simulink model "ofdm_txrx_supermimo_coded"  (Simulink 7.1) (continued)
== system ofdm_txrx_supermimo_coded/EDK Processor == (continued)
      |                                      RNWReg, ...
      |                                      bankAddr, ...
      |                                      xl_slice(linearAddr, ...
      |                                               xl_nbits(linearAddr) - 1, ...
      |                                               5) ...
      |                                     );
      | if opCode_sm_PktBufFreqOffsets == xl_concat(xfix({xlUnsigned, 4, 0}, 8), ...
      |                                          xfix({xlUnsigned, ...
      |                                                xl_nbits(linearAddr) - 5, ...
      |                                                0}, ...
      |                                                66) ...
      |                                         );
      |     sm_PktBufFreqOffsets_we_reg = true;
      | else
      |     sm_PktBufFreqOffsets_we_reg = false;
      | end
      | persistent sm_RxModulation_we_reg; sm_RxModulation_we_reg = xl_state(false, {xlBoolean});
      | sm_RxModulation_we = sm_RxModulation_we_reg;
      | opCode_sm_RxModulation = xl_concat(addrAck, ...
      |                                      RNWReg, ...
      |                                      bankAddr, ...
      |                                      xl_slice(linearAddr, ...
      |                                               xl_nbits(linearAddr) - 1, ...
      |                                               8) ...
      |                                     );
      | if opCode_sm_RxModulation == xl_concat(xfix({xlUnsigned, 4, 0}, 8), ...
      |                                          xfix({xlUnsigned, ...
      |                                                xl_nbits(linearAddr) - 8, ...
      |                                                0}, ...
      |                                                6) ...
      |                                         );
      |     sm_RxModulation_we_reg = true;
      | else
      |     sm_RxModulation_we_reg = false;
      | end
      | persistent sm_TxHeaderTranslate_we_reg; sm_TxHeaderTranslate_we_reg = xl_state(false, {xlBoolean});
      | sm_TxHeaderTranslate_we = sm_TxHeaderTranslate_we_reg;
      | opCode_sm_TxHeaderTranslate = xl_concat(addrAck, ...
      |                                      RNWReg, ...
      |                                      bankAddr, ...
      |                                      xl_slice(linearAddr, ...
      |                                               xl_nbits(linearAddr) - 1, ...
      |                                               10) ...
      |                                     );
      | if opCode_sm_TxHeaderTranslate == xl_concat(xfix({xlUnsigned, 4, 0}, 8), ...
      |                                          xfix({xlUnsigned, ...
      |                                                xl_nbits(linearAddr) - 10, ...
      |                                                0}, ...
      |                                                0) ...
      |                                         );
      |     sm_TxHeaderTranslate_we_reg = true;
      | else
      |     sm_TxHeaderTranslate_we_reg = false;
      | end
      | persistent sm_TxModulation_we_reg; sm_TxModulation_we_reg = xl_state(false, {xlBoolean});
      | sm_TxModulation_we = sm_TxModulation_we_reg;
      | opCode_sm_TxModulation = xl_concat(addrAck, ...
      |                                      RNWReg, ...
      |                                      bankAddr, ...
      |                                      xl_slice(linearAddr, ...
      |                                               xl_nbits(linearAddr) - 1, ...
      |                                               8) ...
      |                                     );
      | if opCode_sm_TxModulation == xl_concat(xfix({xlUnsigned, 4, 0}, 8), ...
      |                                          xfix({xlUnsigned, ...
      |                                                xl_nbits(linearAddr) - 8, ...
      |                                                0}, ...
      |                                                7) ...
      |                                         );
      |     sm_TxModulation_we_reg = true;
      | else
      |     sm_TxModulation_we_reg = false;
      | end
      | 
      | 
      | % 'addr' ports of 'Shared Memory' blocks
      | persistent sm_ChannelEstimates_addr_reg; 
      | sm_ChannelEstimates_addr_reg = xl_state(0, {xlUnsigned, 8, 0});
      | sm_ChannelEstimates_addr = sm_ChannelEstimates_addr_reg;
      | if addrAck == 1
      |     sm_ChannelEstimates_addr_reg = xl_slice(linearAddr, 8, 0);
      | else
      |     sm_ChannelEstimates_addr_reg = sm_ChannelEstimates_addr_reg;
      | end
      | persistent sm_EVM_perSC_addr_reg; 
      | sm_EVM_perSC_addr_reg = xl_state(0, {xlUnsigned, 6, 0});
      | sm_EVM_perSC_addr = sm_EVM_perSC_addr_reg;
      | if addrAck == 1
      |     sm_EVM_perSC_addr_reg = xl_slice(linearAddr, 6, 0);
      | else
      |     sm_EVM_perSC_addr_reg = sm_EVM_perSC_addr_reg;
      | end
      | persistent sm_EVM_perSym_addr_reg; 
      | sm_EVM_perSym_addr_reg = xl_state(0, {xlUnsigned, 8, 0});
      | sm_EVM_perSym_addr = sm_EVM_perSym_addr_reg;
      | if addrAck == 1
      |     sm_EVM_perSym_addr_reg = xl_slice(linearAddr, 8, 0);
      | else
      |     sm_EVM_perSym_addr_reg = sm_EVM_perSym_addr_reg;
      | end
      | persistent sm_PktBufFreqOffsets_addr_reg; 
      | sm_PktBufFreqOffsets_addr_reg = xl_state(0, {xlUnsigned, 5, 0});
      | sm_PktBufFreqOffsets_addr = sm_PktBufFreqOffsets_addr_reg;
      | if addrAck == 1
      |     sm_PktBufFreqOffsets_addr_reg = xl_slice(linearAddr, 5, 0);
      | else
      |     sm_PktBufFreqOffsets_addr_reg = sm_PktBufFreqOffsets_addr_reg;
      | end
      | persistent sm_RxModulation_addr_reg; 
      | sm_RxModulation_addr_reg = xl_state(0, {xlUnsigned, 8, 0});
      | sm_RxModulation_addr = sm_RxModulation_addr_reg;
      | if addrAck == 1
      |     sm_RxModulation_addr_reg = xl_slice(linearAddr, 8, 0);
      | else
      |     sm_RxModulation_addr_reg = sm_RxModulation_addr_reg;
      | end
      | persistent sm_TxHeaderTranslate_addr_reg; 
      | sm_TxHeaderTranslate_addr_reg = xl_state(0, {xlUnsigned, 10, 0});
      | sm_TxHeaderTranslate_addr = sm_TxHeaderTranslate_addr_reg;
      | if addrAck == 1
      |     sm_TxHeaderTranslate_addr_reg = xl_slice(linearAddr, 10, 0);
      | else
      |     sm_TxHeaderTranslate_addr_reg = sm_TxHeaderTranslate_addr_reg;
      | end
      | persistent sm_TxModulation_addr_reg; 
      | sm_TxModulation_addr_reg = xl_state(0, {xlUnsigned, 8, 0});
      | sm_TxModulation_addr = sm_TxModulation_addr_reg;
      | if addrAck == 1
      |     sm_TxModulation_addr_reg = xl_slice(linearAddr, 8, 0);
      | else
      |     sm_TxModulation_addr_reg = sm_TxModulation_addr_reg;
      | end
      | 
      | 
      | % 're' ports of 'From FIFO' blocks
      | 
      | 
      | % 'en' ports of 'To Register' blocks
      | if opCode == xl_concat(xfix({xlUnsigned, 4, 0}, 10), ...
      |                        xfix({xlUnsigned, xl_nbits(linearAddr), 0}, 0))
      |     sm_FEC_Config_w_en = true;
      | else
      |     sm_FEC_Config_w_en = false;
      | end
      | if opCode == xl_concat(xfix({xlUnsigned, 4, 0}, 10), ...
      |                        xfix({xlUnsigned, xl_nbits(linearAddr), 0}, 1))
      |     sm_Rx_AF_Blanking_w_en = true;
      | else
      |     sm_Rx_AF_Blanking_w_en = false;
      | end
      | if opCode == xl_concat(xfix({xlUnsigned, 4, 0}, 10), ...
      |                        xfix({xlUnsigned, xl_nbits(linearAddr), 0}, 2))
      |     sm_Rx_AF_TxScaling_w_en = true;
      | else
      |     sm_Rx_AF_TxScaling_w_en = false;
      | end
      | if opCode == xl_concat(xfix({xlUnsigned, 4, 0}, 10), ...
      |                        xfix({xlUnsigned, xl_nbits(linearAddr), 0}, 3))
      |     sm_Rx_ChanEst_MinMag_w_en = true;
      | else
      |     sm_Rx_ChanEst_MinMag_w_en = false;
      | end
      | if opCode == xl_concat(xfix({xlUnsigned, 4, 0}, 10), ...
      |                        xfix({xlUnsigned, xl_nbits(linearAddr), 0}, 4))
      |     sm_Rx_Constellation_Scaling_w_en = true;
      | else
      |     sm_Rx_Constellation_Scaling_w_en = false;
      | end
      | if opCode == xl_concat(xfix({xlUnsigned, 4, 0}, 10), ...
      |                        xfix({xlUnsigned, xl_nbits(linearAddr), 0}, 5))
      |     sm_Rx_ControlBits_w_en = true;
      | else
      |     sm_Rx_ControlBits_w_en = false;
      | end
      | if opCode == xl_concat(xfix({xlUnsigned, 4, 0}, 10), ...
      |                        xfix({xlUnsigned, xl_nbits(linearAddr), 0}, 6))
      |     sm_Rx_FixedPktLen_w_en = true;
      | else
      |     sm_Rx_FixedPktLen_w_en = false;
      | end
      | if opCode == xl_concat(xfix({xlUnsigned, 4, 0}, 10), ...
      |                        xfix({xlUnsigned, xl_nbits(linearAddr), 0}, 7))
      |     sm_Rx_OFDM_SymbolCounts_w_en = true;
      | else
      |     sm_Rx_OFDM_SymbolCounts_w_en = false;
      | end
      | if opCode == xl_concat(xfix({xlUnsigned, 4, 0}, 10), ...
      |                        xfix({xlUnsigned, xl_nbits(linearAddr), 0}, 8))
      |     sm_Rx_PilotCalcParams_w_en = true;
      | else
      |     sm_Rx_PilotCalcParams_w_en = false;
      | end
      | if opCode == xl_concat(xfix({xlUnsigned, 4, 0}, 10), ...
      |                        xfix({xlUnsigned, xl_nbits(linearAddr), 0}, 9))
      |     sm_Rx_PktDet_Delay_w_en = true;
      | else
      |     sm_Rx_PktDet_Delay_w_en = false;
      | end
      | if opCode == xl_concat(xfix({xlUnsigned, 4, 0}, 10), ...
      |                        xfix({xlUnsigned, xl_nbits(linearAddr), 0}, 10))
      |     sm_Rx_PktDet_LongCorr_Params_w_en = true;
      | else
      |     sm_Rx_PktDet_LongCorr_Params_w_en = false;
      | end
      | if opCode == xl_concat(xfix({xlUnsigned, 4, 0}, 10), ...
      |                        xfix({xlUnsigned, xl_nbits(linearAddr), 0}, 11))
      |     sm_Rx_PktDet_LongCorr_Thresholds_w_en = true;
      | else
      |     sm_Rx_PktDet_LongCorr_Thresholds_w_en = false;
      | end
      | if opCode == xl_concat(xfix({xlUnsigned, 4, 0}, 10), ...
      |                        xfix({xlUnsigned, xl_nbits(linearAddr), 0}, 12))
      |     sm_Rx_PreCFO_Options_w_en = true;
      | else
      |     sm_Rx_PreCFO_Options_w_en = false;
      | end
      | if opCode == xl_concat(xfix({xlUnsigned, 4, 0}, 10), ...
      |                        xfix({xlUnsigned, xl_nbits(linearAddr), 0}, 13))
      |     sm_Rx_PreCFO_PilotCalcCorrection_w_en = true;
      | else
      |     sm_Rx_PreCFO_PilotCalcCorrection_w_en = false;
      | end
      | if opCode == xl_concat(xfix({xlUnsigned, 4, 0}, 10), ...
      |                        xfix({xlUnsigned, xl_nbits(linearAddr), 0}, 14))
      |     sm_Rx_coarseCFO_correction_w_en = true;
      | else
      |     sm_Rx_coarseCFO_correction_w_en = false;
      | end
      | if opCode == xl_concat(xfix({xlUnsigned, 4, 0}, 10), ...
      |                        xfix({xlUnsigned, xl_nbits(linearAddr), 0}, 15))
      |     sm_Rx_pktByteNums_w_en = true;
      | else
      |     sm_Rx_pktByteNums_w_en = false;
      | end
      | if opCode == xl_concat(xfix({xlUnsigned, 4, 0}, 10), ...
      |                        xfix({xlUnsigned, xl_nbits(linearAddr), 0}, 16))
      |     sm_TxRx_AutoReply_Action0_w_en = true;
      | else
      |     sm_TxRx_AutoReply_Action0_w_en = false;
      | end
      | if opCode == xl_concat(xfix({xlUnsigned, 4, 0}, 10), ...
      |                        xfix({xlUnsigned, xl_nbits(linearAddr), 0}, 17))
      |     sm_TxRx_AutoReply_Action1_w_en = true;
      | else
      |     sm_TxRx_AutoReply_Action1_w_en = false;
      | end
      | if opCode == xl_concat(xfix({xlUnsigned, 4, 0}, 10), ...
      |                        xfix({xlUnsigned, xl_nbits(linearAddr), 0}, 18))
      |     sm_TxRx_AutoReply_Action2_w_en = true;
      | else
      |     sm_TxRx_AutoReply_Action2_w_en = false;
      | end
      | if opCode == xl_concat(xfix({xlUnsigned, 4, 0}, 10), ...
      |                        xfix({xlUnsigned, xl_nbits(linearAddr), 0}, 19))
      |     sm_TxRx_AutoReply_Action3_w_en = true;
      | else
      |     sm_TxRx_AutoReply_Action3_w_en = false;
      | end
      | if opCode == xl_concat(xfix({xlUnsigned, 4, 0}, 10), ...
      |                        xfix({xlUnsigned, xl_nbits(linearAddr), 0}, 20))
      |     sm_TxRx_AutoReply_Action4_w_en = true;
      | else
      |     sm_TxRx_AutoReply_Action4_w_en = false;
      | end
      | if opCode == xl_concat(xfix({xlUnsigned, 4, 0}, 10), ...
      |                        xfix({xlUnsigned, xl_nbits(linearAddr), 0}, 21))
      |     sm_TxRx_AutoReply_Action5_w_en = true;
      | else
      |     sm_TxRx_AutoReply_Action5_w_en = false;
      | end
      | if opCode == xl_concat(xfix({xlUnsigned, 4, 0}, 10), ...
      |                        xfix({xlUnsigned, xl_nbits(linearAddr), 0}, 22))
      |     sm_TxRx_AutoReply_Match0_w_en = true;
      | else
      |     sm_TxRx_AutoReply_Match0_w_en = false;
      | end
      | if opCode == xl_concat(xfix({xlUnsigned, 4, 0}, 10), ...
      |                        xfix({xlUnsigned, xl_nbits(linearAddr), 0}, 23))
      |     sm_TxRx_AutoReply_Match1_w_en = true;
      | else
      |     sm_TxRx_AutoReply_Match1_w_en = false;
      | end
      | if opCode == xl_concat(xfix({xlUnsigned, 4, 0}, 10), ...
      |                        xfix({xlUnsigned, xl_nbits(linearAddr), 0}, 24))
      |     sm_TxRx_AutoReply_Match2_w_en = true;
      | else
      |     sm_TxRx_AutoReply_Match2_w_en = false;
      | end
      | if opCode == xl_concat(xfix({xlUnsigned, 4, 0}, 10), ...
      |                        xfix({xlUnsigned, xl_nbits(linearAddr), 0}, 25))
      |     sm_TxRx_AutoReply_Match3_w_en = true;
      | else
      |     sm_TxRx_AutoReply_Match3_w_en = false;
      | end
      | if opCode == xl_concat(xfix({xlUnsigned, 4, 0}, 10), ...
      |                        xfix({xlUnsigned, xl_nbits(linearAddr), 0}, 26))
      |     sm_TxRx_AutoReply_Match4_w_en = true;
      | else
      |     sm_TxRx_AutoReply_Match4_w_en = false;
      | end
      | if opCode == xl_concat(xfix({xlUnsigned, 4, 0}, 10), ...
      |                        xfix({xlUnsigned, xl_nbits(linearAddr), 0}, 27))
      |     sm_TxRx_AutoReply_Match5_w_en = true;
      | else
      |     sm_TxRx_AutoReply_Match5_w_en = false;
      | end
      | if opCode == xl_concat(xfix({xlUnsigned, 4, 0}, 10), ...
      |                        xfix({xlUnsigned, xl_nbits(linearAddr), 0}, 28))
      |     sm_TxRx_AutoReply_Match6_w_en = true;
      | else
      |     sm_TxRx_AutoReply_Match6_w_en = false;
      | end
      | if opCode == xl_concat(xfix({xlUnsigned, 4, 0}, 10), ...
      |                        xfix({xlUnsigned, xl_nbits(linearAddr), 0}, 29))
      |     sm_TxRx_AutoReply_Match7_w_en = true;
      | else
      |     sm_TxRx_AutoReply_Match7_w_en = false;
      | end
      | if opCode == xl_concat(xfix({xlUnsigned, 4, 0}, 10), ...
      |                        xfix({xlUnsigned, xl_nbits(linearAddr), 0}, 30))
      |     sm_TxRx_FFT_Scaling_w_en = true;
      | else
      |     sm_TxRx_FFT_Scaling_w_en = false;
      | end
      | if opCode == xl_concat(xfix({xlUnsigned, 4, 0}, 10), ...
      |                        xfix({xlUnsigned, xl_nbits(linearAddr), 0}, 31))
      |     sm_TxRx_Interrupt_PktBuf_Ctrl_w_en = true;
      | else
      |     sm_TxRx_Interrupt_PktBuf_Ctrl_w_en = false;
      | end
      | if opCode == xl_concat(xfix({xlUnsigned, 4, 0}, 10), ...
      |                        xfix({xlUnsigned, xl_nbits(linearAddr), 0}, 32))
      |     sm_TxRx_Pilots_Index_w_en = true;
      | else
      |     sm_TxRx_Pilots_Index_w_en = false;
      | end
      | if opCode == xl_concat(xfix({xlUnsigned, 4, 0}, 10), ...
      |                        xfix({xlUnsigned, xl_nbits(linearAddr), 0}, 33))
      |     sm_TxRx_Pilots_Values_w_en = true;
      | else
      |     sm_TxRx_Pilots_Values_w_en = false;
      | end
      | if opCode == xl_concat(xfix({xlUnsigned, 4, 0}, 10), ...
      |                        xfix({xlUnsigned, xl_nbits(linearAddr), 0}, 34))
      |     sm_Tx_ControlBits_w_en = true;
      | else
      |     sm_Tx_ControlBits_w_en = false;
      | end
      | if opCode == xl_concat(xfix({xlUnsigned, 4, 0}, 10), ...
      |                        xfix({xlUnsigned, xl_nbits(linearAddr), 0}, 35))
      |     sm_Tx_Delays_w_en = true;
      | else
      |     sm_Tx_Delays_w_en = false;
      | end
      | if opCode == xl_concat(xfix({xlUnsigned, 4, 0}, 10), ...
      |                        xfix({xlUnsigned, xl_nbits(linearAddr), 0}, 36))
      |     sm_Tx_OFDM_SymCounts_w_en = true;
      | else
      |     sm_Tx_OFDM_SymCounts_w_en = false;
      | end
      | if opCode == xl_concat(xfix({xlUnsigned, 4, 0}, 10), ...
      |                        xfix({xlUnsigned, xl_nbits(linearAddr), 0}, 37))
      |     sm_Tx_Scaling_w_en = true;
      | else
      |     sm_Tx_Scaling_w_en = false;
      | end
      | if opCode == xl_concat(xfix({xlUnsigned, 4, 0}, 10), ...
      |                        xfix({xlUnsigned, xl_nbits(linearAddr), 0}, 38))
      |     sm_Tx_Start_Reset_Control_w_en = true;
      | else
      |     sm_Tx_Start_Reset_Control_w_en = false;
      | end
      | if opCode == xl_concat(xfix({xlUnsigned, 4, 0}, 10), ...
      |                        xfix({xlUnsigned, xl_nbits(linearAddr), 0}, 39))
      |     sm_pktDet_autoCorrParams_w_en = true;
      | else
      |     sm_pktDet_autoCorrParams_w_en = false;
      | end
      | if opCode == xl_concat(xfix({xlUnsigned, 4, 0}, 10), ...
      |                        xfix({xlUnsigned, xl_nbits(linearAddr), 0}, 40))
      |     sm_pktDet_controlBits_w_en = true;
      | else
      |     sm_pktDet_controlBits_w_en = false;
      | end
      | if opCode == xl_concat(xfix({xlUnsigned, 4, 0}, 10), ...
      |                        xfix({xlUnsigned, xl_nbits(linearAddr), 0}, 41))
      |     sm_pktDet_durations_w_en = true;
      | else
      |     sm_pktDet_durations_w_en = false;
      | end
      | if opCode == xl_concat(xfix({xlUnsigned, 4, 0}, 10), ...
      |                        xfix({xlUnsigned, xl_nbits(linearAddr), 0}, 42))
      |     sm_pktDet_thresholds_w_en = true;
      | else
      |     sm_pktDet_thresholds_w_en = false;
      | end
      | 
      | 
      | % 'din' ports of 'To FIFO' blocks
      | 
      | 
      | % 'we' ports of 'To FIFO' blocks
      | 
      | 
      | % 'din' ports of 'To Register' blocks
      | sm_FEC_Config_w_din = xl_force(xl_slice(wrDBus, 32 - 1, 0), ...
      |                                  xlUnsigned, ...
      |                                  0);
      | sm_Rx_AF_Blanking_w_din = xl_force(xl_slice(wrDBus, 32 - 1, 0), ...
      |                                  xlUnsigned, ...
      |                                  0);
      | sm_Rx_AF_TxScaling_w_din = xl_force(xl_slice(wrDBus, 32 - 1, 0), ...
      |                                  xlUnsigned, ...
      |                                  0);
      | sm_Rx_ChanEst_MinMag_w_din = xl_force(xl_slice(wrDBus, 32 - 1, 0), ...
      |                                  xlUnsigned, ...
      |                                  0);
      | sm_Rx_Constellation_Scaling_w_din = xl_force(xl_slice(wrDBus, 32 - 1, 0), ...
      |                                  xlUnsigned, ...
      |                                  0);
      | sm_Rx_ControlBits_w_din = xl_force(xl_slice(wrDBus, 32 - 1, 0), ...
      |                                  xlUnsigned, ...
      |                                  0);
      | sm_Rx_FixedPktLen_w_din = xl_force(xl_slice(wrDBus, 32 - 1, 0), ...
      |                                  xlUnsigned, ...
      |                                  0);
      | sm_Rx_OFDM_SymbolCounts_w_din = xl_force(xl_slice(wrDBus, 32 - 1, 0), ...
      |                                  xlUnsigned, ...
      |                                  0);
      | sm_Rx_PilotCalcParams_w_din = xl_force(xl_slice(wrDBus, 32 - 1, 0), ...
      |                                  xlUnsigned, ...
      |                                  0);
      | sm_Rx_PktDet_Delay_w_din = xl_force(xl_slice(wrDBus, 32 - 1, 0), ...
      |                                  xlUnsigned, ...
      |                                  0);
      | sm_Rx_PktDet_LongCorr_Params_w_din = xl_force(xl_slice(wrDBus, 32 - 1, 0), ...
      |                                  xlUnsigned, ...
      |                                  0);
      | sm_Rx_PktDet_LongCorr_Thresholds_w_din = xl_force(xl_slice(wrDBus, 32 - 1, 0), ...
      |                                  xlUnsigned, ...
      |                                  0);
      | sm_Rx_PreCFO_Options_w_din = xl_force(xl_slice(wrDBus, 32 - 1, 0), ...
      |                                  xlUnsigned, ...
      |                                  0);
      | sm_Rx_PreCFO_PilotCalcCorrection_w_din = xl_force(xl_slice(wrDBus, 32 - 1, 0), ...
      |                                  xlUnsigned, ...
      |                                  0);
      | sm_Rx_coarseCFO_correction_w_din = xl_force(xl_slice(wrDBus, 32 - 1, 0), ...
      |                                  xlUnsigned, ...
      |                                  0);
      | sm_Rx_pktByteNums_w_din = xl_force(xl_slice(wrDBus, 32 - 1, 0), ...
      |                                  xlUnsigned, ...
      |                                  0);
      | sm_TxRx_AutoReply_Action0_w_din = xl_force(xl_slice(wrDBus, 32 - 1, 0), ...
      |                                  xlUnsigned, ...
      |                                  0);
      | sm_TxRx_AutoReply_Action1_w_din = xl_force(xl_slice(wrDBus, 32 - 1, 0), ...
      |                                  xlUnsigned, ...
      |                                  0);
      | sm_TxRx_AutoReply_Action2_w_din = xl_force(xl_slice(wrDBus, 32 - 1, 0), ...
      |                                  xlUnsigned, ...
      |                                  0);
      | sm_TxRx_AutoReply_Action3_w_din = xl_force(xl_slice(wrDBus, 32 - 1, 0), ...
      |                                  xlUnsigned, ...
      |                                  0);
      | sm_TxRx_AutoReply_Action4_w_din = xl_force(xl_slice(wrDBus, 32 - 1, 0), ...
      |                                  xlUnsigned, ...
      |                                  0);
      | sm_TxRx_AutoReply_Action5_w_din = xl_force(xl_slice(wrDBus, 32 - 1, 0), ...
      |                                  xlUnsigned, ...
      |                                  0);
      | sm_TxRx_AutoReply_Match0_w_din = xl_force(xl_slice(wrDBus, 32 - 1, 0), ...
      |                                  xlUnsigned, ...
      |                                  0);
      | sm_TxRx_AutoReply_Match1_w_din = xl_force(xl_slice(wrDBus, 32 - 1, 0), ...
      |                                  xlUnsigned, ...
      |                                  0);
      | sm_TxRx_AutoReply_Match2_w_din = xl_force(xl_slice(wrDBus, 32 - 1, 0), ...
      |                                  xlUnsigned, ...
      |                                  0);
      | sm_TxRx_AutoReply_Match3_w_din = xl_force(xl_slice(wrDBus, 32 - 1, 0), ...
      |                                  xlUnsigned, ...
      |                                  0);
      | sm_TxRx_AutoReply_Match4_w_din = xl_force(xl_slice(wrDBus, 32 - 1, 0), ...
      |                                  xlUnsigned, ...
      |                                  0);
      | sm_TxRx_AutoReply_Match5_w_din = xl_force(xl_slice(wrDBus, 32 - 1, 0), ...
      |                                  xlUnsigned, ...
      |                                  0);
      | sm_TxRx_AutoReply_Match6_w_din = xl_force(xl_slice(wrDBus, 32 - 1, 0), ...
      |                                  xlUnsigned, ...
      |                                  0);
      | sm_TxRx_AutoReply_Match7_w_din = xl_force(xl_slice(wrDBus, 32 - 1, 0), ...
      |                                  xlUnsigned, ...
      |                                  0);
      | sm_TxRx_FFT_Scaling_w_din = xl_force(xl_slice(wrDBus, 12 - 1, 0), ...
      |                                  xlUnsigned, ...
      |                                  0);
      | sm_TxRx_Interrupt_PktBuf_Ctrl_w_din = xl_force(xl_slice(wrDBus, 32 - 1, 0), ...
      |                                  xlUnsigned, ...
      |                                  0);
      | sm_TxRx_Pilots_Index_w_din = xl_force(xl_slice(wrDBus, 32 - 1, 0), ...
      |                                  xlUnsigned, ...
      |                                  0);
      | sm_TxRx_Pilots_Values_w_din = xl_force(xl_slice(wrDBus, 32 - 1, 0), ...
      |                                  xlUnsigned, ...
      |                                  0);
      | sm_Tx_ControlBits_w_din = xl_force(xl_slice(wrDBus, 32 - 1, 0), ...
      |                                  xlUnsigned, ...
      |                                  0);
      | sm_Tx_Delays_w_din = xl_force(xl_slice(wrDBus, 32 - 1, 0), ...
      |                                  xlUnsigned, ...
      |                                  0);
      | sm_Tx_OFDM_SymCounts_w_din = xl_force(xl_slice(wrDBus, 32 - 1, 0), ...
      |                                  xlUnsigned, ...
      |                                  0);
      | sm_Tx_Scaling_w_din = xl_force(xl_slice(wrDBus, 32 - 1, 0), ...
      |                                  xlUnsigned, ...
      |                                  0);
      | sm_Tx_Start_Reset_Control_w_din = xl_force(xl_slice(wrDBus, 32 - 1, 0), ...
      |                                  xlUnsigned, ...
      |                                  0);
      | sm_pktDet_autoCorrParams_w_din = xl_force(xl_slice(wrDBus, 32 - 1, 0), ...
      |                                  xlUnsigned, ...
      |                                  0);
      | sm_pktDet_controlBits_w_din = xl_force(xl_slice(wrDBus, 32 - 1, 0), ...
      |                                  xlUnsigned, ...
      |                                  0);
      | sm_pktDet_durations_w_din = xl_force(xl_slice(wrDBus, 32 - 1, 0), ...
      |                                  xlUnsigned, ...
      |                                  0);
      | sm_pktDet_thresholds_w_din = xl_force(xl_slice(wrDBus, 32 - 1, 0), ...
      |                                  xlUnsigned, ...
      |                                  0);
      | 
      | 
      | persistent read_bank_out_reg; read_bank_out_reg = xl_state(0, {xlUnsigned, 32, 0});
      | read_bank_out = read_bank_out_reg;
      | 
      | persistent bankAddr_reg; bankAddr_reg = xl_state(0, bankAddr);
      | 
      | if bankAddr_reg == 0
      |     % Bank 0: Shared Memories
      |     read_bank_out_reg = ram_bank_out;
      | elseif bankAddr_reg == 1
      |     % Bank 1: From/To FIFOs
      |     read_bank_out_reg =  0;
      | elseif bankAddr_reg == 2
      |     % Bank 2: From/To Registers
      |     read_bank_out_reg = reg_bank_out;
      | elseif bankAddr_reg == 3
      |     % Bank 3: Configuration Registers
      |     read_bank_out_reg = 0;
      | end
      | 
      | bankAddr_reg = bankAddr;
      settings: block_version=10.1.3
  sg_plb_addrpref : xbsIndex_r4/Gateway In  arith_type=Unsigned  n_bits=16  bin_pt=0  quantization="Round  (unbiased: +/- Inf)"  overflow=Saturate  period=xlGetSimulinkPeriod(gcb)  dbl_ovrd=off  timing_constraint=None  locs_specified=off  LOCs={}  block_type=gatewayin
      settings: infoedit="Gateway in block.  Converts inputs of type Simulink integer, double and fixed point to  Xilinx fixed point type.<P><P>Hardware notes:  In hardware these blocks become top level input ports."  sginterface={'Xilinx'=>{'jtaghwcosim'=>{'non_memory_mapped_port'=>'sg_plb_addrpref'}},'iopad'=>{'constraint'=>'#'}}  block_version=10.1.3
  wires:
  plb_decode/2 -> plb_memmap/5, Sl_addrAck/1  "Sl_addrAck"
  plb_decode/4 -> Sl_wrDAck/1, Sl_wrComp/1  "Sl_wrDAck"
  plb_memmap/108 -> Shared Memory6/3  "TxModulation_we"
  plb_memmap/107 -> Shared Memory6/2  "TxModulation_din"
  plb_memmap/106 -> Shared Memory6/1  "TxModulation_addr"
  plb_memmap/105 -> Shared Memory5/3  "TxHeaderTranslate_we"
  plb_memmap/104 -> Shared Memory5/2  "TxHeaderTranslate_din"
  plb_memmap/103 -> Shared Memory5/1  "TxHeaderTranslate_addr"
  plb_memmap/102 -> Shared Memory4/3  "RxModulation_we"
  plb_memmap/101 -> Shared Memory4/2  "RxModulation_din"
  plb_memmap/100 -> Shared Memory4/1  "RxModulation_addr"
  plb_memmap/99 -> Shared Memory3/3  "PktBufFreqOffsets_we"
  plb_memmap/98 -> Shared Memory3/2  "PktBufFreqOffsets_din"
  plb_memmap/97 -> Shared Memory3/1  "PktBufFreqOffsets_addr"
  plb_memmap/96 -> Shared Memory2/3  "EVM_perSym_we"
  plb_memmap/95 -> Shared Memory2/2  "EVM_perSym_din"
  plb_memmap/94 -> Shared Memory2/1  "EVM_perSym_addr"
  plb_memmap/93 -> Shared Memory1/3  "EVM_perSC_we"
  plb_memmap/92 -> Shared Memory1/2  "EVM_perSC_din"
  plb_memmap/91 -> Shared Memory1/1  "EVM_perSC_addr"
  plb_memmap/90 -> Shared Memory/3  "ChannelEstimates_we"
  plb_memmap/89 -> Shared Memory/2  "ChannelEstimates_din"
  plb_memmap/88 -> Shared Memory/1  "ChannelEstimates_addr"
  plb_memmap/87 -> To Register42/2  "pktDet_thresholds_w_en"
  plb_memmap/86 -> To Register42/1  "pktDet_thresholds_w_din"
  plb_memmap/85 -> To Register41/2  "pktDet_durations_w_en"
  plb_memmap/84 -> To Register41/1  "pktDet_durations_w_din"
  plb_memmap/83 -> To Register40/2  "pktDet_controlBits_w_en"
  plb_memmap/82 -> To Register40/1  "pktDet_controlBits_w_din"
  plb_memmap/81 -> To Register39/2  "pktDet_autoCorrParams_w_en"
  plb_memmap/80 -> To Register39/1  "pktDet_autoCorrParams_w_din"
  plb_memmap/79 -> To Register38/2  "Tx_Start_Reset_Control_w_en"
  plb_memmap/78 -> To Register38/1  "Tx_Start_Reset_Control_w_din"
  plb_memmap/77 -> To Register37/2  "Tx_Scaling_w_en"
  plb_memmap/76 -> To Register37/1  "Tx_Scaling_w_din"
  plb_memmap/75 -> To Register36/2  "Tx_OFDM_SymCounts_w_en"
  plb_memmap/74 -> To Register36/1  "Tx_OFDM_SymCounts_w_din"
  plb_memmap/73 -> To Register35/2  "Tx_Delays_w_en"
  plb_memmap/72 -> To Register35/1  "Tx_Delays_w_din"
  plb_memmap/71 -> To Register34/2  "Tx_ControlBits_w_en"
  plb_memmap/70 -> To Register34/1  "Tx_ControlBits_w_din"
  plb_memmap/69 -> To Register33/2  "TxRx_Pilots_Values_w_en"
  plb_memmap/68 -> To Register33/1  "TxRx_Pilots_Values_w_din"
  plb_memmap/67 -> To Register32/2  "TxRx_Pilots_Index_w_en"
  plb_memmap/66 -> To Register32/1  "TxRx_Pilots_Index_w_din"
  plb_memmap/65 -> To Register31/2  "TxRx_Interrupt_PktBuf_Ctrl_w_en"
  plb_memmap/64 -> To Register31/1  "TxRx_Interrupt_PktBuf_Ctrl_w_din"
  plb_memmap/63 -> To Register30/2  "TxRx_FFT_Scaling_w_en"
  plb_memmap/62 -> To Register30/1  "TxRx_FFT_Scaling_w_din"
  plb_memmap/61 -> To Register29/2  "TxRx_AutoReply_Match7_w_en"
  plb_memmap/60 -> To Register29/1  "TxRx_AutoReply_Match7_w_din"
  plb_memmap/59 -> To Register28/2  "TxRx_AutoReply_Match6_w_en"
  plb_memmap/58 -> To Register28/1  "TxRx_AutoReply_Match6_w_din"
  plb_memmap/57 -> To Register27/2  "TxRx_AutoReply_Match5_w_en"
  plb_memmap/56 -> To Register27/1  "TxRx_AutoReply_Match5_w_din"
  plb_memmap/55 -> To Register26/2  "TxRx_AutoReply_Match4_w_en"
  plb_memmap/54 -> To Register26/1  "TxRx_AutoReply_Match4_w_din"
  plb_memmap/53 -> To Register25/2  "TxRx_AutoReply_Match3_w_en"
  plb_memmap/52 -> To Register25/1  "TxRx_AutoReply_Match3_w_din"
  plb_memmap/51 -> To Register24/2  "TxRx_AutoReply_Match2_w_en"
  plb_memmap/50 -> To Register24/1  "TxRx_AutoReply_Match2_w_din"
  plb_memmap/49 -> To Register23/2  "TxRx_AutoReply_Match1_w_en"
  plb_memmap/48 -> To Register23/1  "TxRx_AutoReply_Match1_w_din"
  plb_memmap/47 -> To Register22/2  "TxRx_AutoReply_Match0_w_en"
  plb_memmap/46 -> To Register22/1  "TxRx_AutoReply_Match0_w_din"
  plb_memmap/45 -> To Register21/2  "TxRx_AutoReply_Action5_w_en"
  plb_memmap/44 -> To Register21/1  "TxRx_AutoReply_Action5_w_din"
  plb_memmap/43 -> To Register20/2  "TxRx_AutoReply_Action4_w_en"
  plb_memmap/42 -> To Register20/1  "TxRx_AutoReply_Action4_w_din"
  plb_memmap/41 -> To Register19/2  "TxRx_AutoReply_Action3_w_en"
  plb_memmap/40 -> To Register19/1  "TxRx_AutoReply_Action3_w_din"
  plb_memmap/39 -> To Register18/2  "TxRx_AutoReply_Action2_w_en"
  plb_memmap/38 -> To Register18/1  "TxRx_AutoReply_Action2_w_din"
  plb_memmap/37 -> To Register17/2  "TxRx_AutoReply_Action1_w_en"
  plb_memmap/36 -> To Register17/1  "TxRx_AutoReply_Action1_w_din"
  plb_memmap/35 -> To Register16/2  "TxRx_AutoReply_Action0_w_en"
  plb_memmap/34 -> To Register16/1  "TxRx_AutoReply_Action0_w_din"
  plb_memmap/33 -> To Register15/2  "Rx_pktByteNums_w_en"
  plb_memmap/32 -> To Register15/1  "Rx_pktByteNums_w_din"
  plb_memmap/31 -> To Register14/2  "Rx_coarseCFO_correction_w_en"
  plb_memmap/30 -> To Register14/1  "Rx_coarseCFO_correction_w_din"
  plb_memmap/29 -> To Register13/2  "Rx_PreCFO_PilotCalcCorrection_w_en"
  plb_memmap/28 -> To Register13/1  "Rx_PreCFO_PilotCalcCorrection_w_din"
  plb_memmap/27 -> To Register12/2  "Rx_PreCFO_Options_w_en"
  plb_memmap/26 -> To Register12/1  "Rx_PreCFO_Options_w_din"
  plb_memmap/25 -> To Register11/2  "Rx_PktDet_LongCorr_Thresholds_w_en"
  plb_memmap/24 -> To Register11/1  "Rx_PktDet_LongCorr_Thresholds_w_din"
  plb_memmap/23 -> To Register10/2  "Rx_PktDet_LongCorr_Params_w_en"
  plb_memmap/22 -> To Register10/1  "Rx_PktDet_LongCorr_Params_w_din"
  plb_memmap/21 -> To Register9/2  "Rx_PktDet_Delay_w_en"
  plb_memmap/20 -> To Register9/1  "Rx_PktDet_Delay_w_din"
  plb_memmap/19 -> To Register8/2  "Rx_PilotCalcParams_w_en"
  plb_memmap/18 -> To Register8/1  "Rx_PilotCalcParams_w_din"
  plb_memmap/17 -> To Register7/2  "Rx_OFDM_SymbolCounts_w_en"
  plb_memmap/16 -> To Register7/1  "Rx_OFDM_SymbolCounts_w_din"
  plb_memmap/15 -> To Register6/2  "Rx_FixedPktLen_w_en"
  plb_memmap/14 -> To Register6/1  "Rx_FixedPktLen_w_din"
  plb_memmap/13 -> To Register5/2  "Rx_ControlBits_w_en"
  plb_memmap/12 -> To Register5/1  "Rx_ControlBits_w_din"
  plb_memmap/11 -> To Register4/2  "Rx_Constellation_Scaling_w_en"
  plb_memmap/10 -> To Register4/1  "Rx_Constellation_Scaling_w_din"
  plb_memmap/9 -> To Register3/2  "Rx_ChanEst_MinMag_w_en"
  plb_memmap/8 -> To Register3/1  "Rx_ChanEst_MinMag_w_din"
  plb_memmap/7 -> To Register2/2  "Rx_AF_TxScaling_w_en"
  plb_memmap/6 -> To Register2/1  "Rx_AF_TxScaling_w_din"
  plb_memmap/5 -> To Register1/2  "Rx_AF_Blanking_w_en"
  plb_memmap/4 -> To Register1/1  "Rx_AF_Blanking_w_din"
  plb_memmap/3 -> To Register/2  "FEC_Config_w_en"
  plb_memmap/2 -> To Register/1  "FEC_Config_w_din"
  plb_memmap/1 -> plb_decode/6  "rdData"
  Shared Memory6/1 -> plb_memmap/50  "TxModulation_dout"
  Shared Memory5/1 -> plb_memmap/49  "TxHeaderTranslate_dout"
  Shared Memory4/1 -> plb_memmap/48  "RxModulation_dout"
  Shared Memory3/1 -> plb_memmap/47  "PktBufFreqOffsets_dout"
  Shared Memory2/1 -> plb_memmap/46  "EVM_perSym_dout"
  Shared Memory1/1 -> plb_memmap/45  "EVM_perSC_dout"
  Shared Memory/1 -> plb_memmap/44  "ChannelEstimates_dout"
  From Register37/1 -> plb_memmap/43  "pktDet_thresholds_r_dout"
  From Register36/1 -> plb_memmap/42  "pktDet_status_dout"
  From Register35/1 -> plb_memmap/41  "pktDet_durations_r_dout"
  From Register34/1 -> plb_memmap/40  "pktDet_controlBits_r_dout"
  From Register33/1 -> plb_memmap/39  "pktDet_autoCorrParams_r_dout"
  From Register32/1 -> plb_memmap/38  "midPacketRSSI_dout"
  From Register31/1 -> plb_memmap/37  "Tx_Start_Reset_Control_r_dout"
  From Register30/1 -> plb_memmap/36  "Tx_PktRunning_dout"
  From Register29/1 -> plb_memmap/35  "Tx_OFDM_SymCounts_r_dout"
  From Register28/1 -> plb_memmap/34  "Tx_Delays_r_dout"
  From Register27/1 -> plb_memmap/33  "Tx_ControlBits_r_dout"
  From Register26/1 -> plb_memmap/32  "TxRx_Interrupt_PktBuf_Ctrl_r_dout"
  From Register25/1 -> plb_memmap/31  "TxRx_AutoReply_Match7_r_dout"
  From Register24/1 -> plb_memmap/30  "TxRx_AutoReply_Match6_r_dout"
  From Register23/1 -> plb_memmap/29  "TxRx_AutoReply_Match5_r_dout"
  From Register22/1 -> plb_memmap/28  "TxRx_AutoReply_Match4_r_dout"
  From Register21/1 -> plb_memmap/27  "TxRx_AutoReply_Match3_r_dout"
  From Register20/1 -> plb_memmap/26  "TxRx_AutoReply_Match2_r_dout"
  From Register19/1 -> plb_memmap/25  "TxRx_AutoReply_Match1_r_dout"
  From Register18/1 -> plb_memmap/24  "TxRx_AutoReply_Match0_r_dout"
  From Register17/1 -> plb_memmap/23  "TxRx_AutoReply_Action5_r_dout"
  From Register16/1 -> plb_memmap/22  "TxRx_AutoReply_Action4_r_dout"
  From Register15/1 -> plb_memmap/21  "TxRx_AutoReply_Action3_r_dout"
  From Register14/1 -> plb_memmap/20  "TxRx_AutoReply_Action2_r_dout"
  From Register13/1 -> plb_memmap/19  "TxRx_AutoReply_Action1_r_dout"
  From Register12/1 -> plb_memmap/18  "TxRx_AutoReply_Action0_r_dout"
  From Register11/1 -> plb_memmap/17  "Rx_pktDone_interruptStatus_dout"
  From Register10/1 -> plb_memmap/16  "Rx_pktDetEventCount_dout"
  From Register9/1 -> plb_memmap/15  "Rx_pktByteNums_r_dout"
  From Register8/1 -> plb_memmap/14  "Rx_pilotCFOest_dout"
  From Register7/1 -> plb_memmap/13  "Rx_coarseCFOest_dout"
  From Register6/1 -> plb_memmap/12  "Rx_PktDet_LongCorr_Params_r_dout"
  From Register5/1 -> plb_memmap/11  "Rx_PktDet_Delay_r_dout"
  From Register4/1 -> plb_memmap/10  "Rx_OFDM_SymbolCounts_r_dout"
  From Register3/1 -> plb_memmap/9  "Rx_Gains_dout"
  From Register2/1 -> plb_memmap/8  "Rx_ControlBits_r_dout"
  From Register1/1 -> plb_memmap/7  "Rx_BER_TotalBits_dout"
  From Register/1 -> plb_memmap/6  "Rx_BER_Errors_dout"
  plb_decode/6 -> plb_memmap/4  "RNWReg"
  plb_decode/9 -> plb_memmap/3  "linearAddr"
  plb_decode/5 -> plb_memmap/2  "bankAddr"
  plb_decode/1 -> plb_memmap/1  "wrDBusReg"
  To Register42/1 -> Terminator49/1  "pktDet_thresholds_w_dout"
  To Register41/1 -> Terminator48/1  "pktDet_durations_w_dout"
  To Register40/1 -> Terminator47/1  "pktDet_controlBits_w_dout"
  To Register39/1 -> Terminator46/1  "pktDet_autoCorrParams_w_dout"
  To Register38/1 -> Terminator45/1  "Tx_Start_Reset_Control_w_dout"
  To Register37/1 -> Terminator44/1  "Tx_Scaling_w_dout"
  To Register36/1 -> Terminator43/1  "Tx_OFDM_SymCounts_w_dout"
  To Register35/1 -> Terminator42/1  "Tx_Delays_w_dout"
  To Register34/1 -> Terminator41/1  "Tx_ControlBits_w_dout"
  To Register33/1 -> Terminator40/1  "TxRx_Pilots_Values_w_dout"
  To Register32/1 -> Terminator39/1  "TxRx_Pilots_Index_w_dout"
  To Register31/1 -> Terminator38/1  "TxRx_Interrupt_PktBuf_Ctrl_w_dout"
  To Register30/1 -> Terminator37/1  "TxRx_FFT_Scaling_w_dout"
  To Register29/1 -> Terminator36/1  "TxRx_AutoReply_Match7_w_dout"
  To Register28/1 -> Terminator35/1  "TxRx_AutoReply_Match6_w_dout"
  To Register27/1 -> Terminator34/1  "TxRx_AutoReply_Match5_w_dout"
  To Register26/1 -> Terminator33/1  "TxRx_AutoReply_Match4_w_dout"
  To Register25/1 -> Terminator32/1  "TxRx_AutoReply_Match3_w_dout"
  To Register24/1 -> Terminator31/1  "TxRx_AutoReply_Match2_w_dout"
  To Register23/1 -> Terminator30/1  "TxRx_AutoReply_Match1_w_dout"
  To Register22/1 -> Terminator29/1  "TxRx_AutoReply_Match0_w_dout"
  To Register21/1 -> Terminator28/1  "TxRx_AutoReply_Action5_w_dout"
  To Register20/1 -> Terminator27/1  "TxRx_AutoReply_Action4_w_dout"
  To Register19/1 -> Terminator26/1  "TxRx_AutoReply_Action3_w_dout"
  To Register18/1 -> Terminator25/1  "TxRx_AutoReply_Action2_w_dout"
  To Register17/1 -> Terminator24/1  "TxRx_AutoReply_Action1_w_dout"
  To Register16/1 -> Terminator23/1  "TxRx_AutoReply_Action0_w_dout"
  To Register15/1 -> Terminator22/1  "Rx_pktByteNums_w_dout"
  To Register14/1 -> Terminator21/1  "Rx_coarseCFO_correction_w_dout"
  To Register13/1 -> Terminator20/1  "Rx_PreCFO_PilotCalcCorrection_w_dout"
  To Register12/1 -> Terminator19/1  "Rx_PreCFO_Options_w_dout"
  To Register11/1 -> Terminator18/1  "Rx_PktDet_LongCorr_Thresholds_w_dout"
  To Register10/1 -> Terminator17/1  "Rx_PktDet_LongCorr_Params_w_dout"
  To Register9/1 -> Terminator16/1  "Rx_PktDet_Delay_w_dout"
  To Register8/1 -> Terminator15/1  "Rx_PilotCalcParams_w_dout"
  To Register7/1 -> Terminator14/1  "Rx_OFDM_SymbolCounts_w_dout"
  To Register6/1 -> Terminator13/1  "Rx_FixedPktLen_w_dout"
  To Register5/1 -> Terminator12/1  "Rx_ControlBits_w_dout"
  To Register4/1 -> Terminator11/1  "Rx_Constellation_Scaling_w_dout"
  To Register3/1 -> Terminator10/1  "Rx_ChanEst_MinMag_w_dout"
  To Register2/1 -> Terminator9/1  "Rx_AF_TxScaling_w_dout"
  To Register1/1 -> Terminator8/1  "Rx_AF_Blanking_w_dout"
  To Register/1 -> Terminator7/1  "FEC_Config_w_dout"
  plb_decode/8 -> Sl_rdDBus/1  "Sl_rdDBus"
  plb_decode/7 -> Sl_rdDAck/1  "Sl_rdDAck"
  plb_decode/3 -> Sl_rdComp/1  "Sl_rdComp"
  sg_plb_addrpref/1 -> plb_decode/7  "addrPref"
  PLB_wrDBus/1 -> plb_decode/5  "PLB_wrDBus"
  PLB_RNW/1 -> plb_decode/4  "PLB_RNW"
  PLB_PAValid/1 -> plb_decode/3  "PLB_PAValid"
  PLB_ABus/1 -> plb_decode/2  "PLB_ABus"
  SPLB_Rst/1 -> plb_decode/1  "SPLB_Rst"
  Constant6/1 -> sg_plb_addrpref/1
  Constant5/1 -> Sl_wait/1  "Sl_wait"
  Constant4/1 -> PLB_wrDBus/1
  Constant3/1 -> PLB_RNW/1
  Constant2/1 -> PLB_PAValid/1
  Constant1/1 -> PLB_ABus/1
  Constant/1 -> SPLB_Rst/1
  Sl_wrComp/1 -> Terminator6/1
  Sl_wrDAck/1 -> Terminator5/1
  Sl_wait/1 -> Terminator4/1
  Sl_rdDBus/1 -> Terminator3/1
  Sl_rdDAck/1 -> Terminator2/1
  Sl_rdComp/1 -> Terminator1/1
  Sl_addrAck/1 -> Terminator/1
